simulink model "ofdm_txrx_mimo_2x"  (Simulink 6.6) (continued)
== system ofdm_txrx_mimo_2x/EDK Processor == (continued)
      | 
      | ps = xl_and(ps1Reg, aValidReg);
      | 
      | % ===== addrAck =====
      | 
      | % register ps
      | persistent psReg, psReg = xl_state(0, ps);
      | 
      | addrAck = xfix({xlUnsigned, 1, 0}, xl_and(xl_not(plbRstReg_), ps, xl_not(psReg)));
      | 
      | psReg = ps;
      | 
      | % ===== rdComp, rd/wr DAck =====
      |  
      | rdComp1 = xfix({xlUnsigned, 1, 0}, xl_and(addrAck, RNWReg));
      | 
      | NUM_rdCompDelay = 3;
      | persistent rdCompDelay, rdCompDelay = xl_state(zeros(1, NUM_rdCompDelay), rdComp1, NUM_rdCompDelay);
      | rdComp2 = rdCompDelay.back;
      | rdCompDelay.push_front_pop_back(rdComp1);
      | 
      | persistent rdCompReg, rdCompReg = xl_state(0, rdComp1);
      | rdComp = rdCompReg;
      | rdCompReg = rdComp2;
      | 
      | persistent rdDAckReg, rdDAckReg = xl_state(0, rdComp1);
      | rdDAck = rdDAckReg;
      | rdDAckReg = rdComp;
      | 
      | persistent wrDAckReg, wrDAckReg = xl_state(0, addrAck);
      | wrDAck = wrDAckReg;
      | wrDAckReg = xl_and(addrAck, xl_not(RNWReg));
      | 
      | % ===== rdDBus =====
      | 
      | rdSel = xl_or(rdComp2, rdComp);
      | 
      | if rdSel == 1
      |     rdDBus1 = rdData;
      | else
      |     rdDBus1 = 0;
      | end % if
      | 
      | persistent rdDBusReg, rdDBusReg = xl_state(0, rdDBus1);
      | rdDBus = rdDBusReg;
      | rdDBusReg = rdDBus1;
      | 
      | % rdDBus = xl_concat(rdDBus32, rdDBus32);
      | % rdDBus = rdDBus32;
      | 
      | % ===== update the persistent variables =====
      | 
      | plbRstReg_ = plbRst;
      | plbABusReg_ = plbABus;
      | plbPAValidReg_ = plbPAValid;
      | plbRNWReg_ = plbRNW;
      | plbWrDBusReg_ = xl_slice(plbWrDBus, DBUS_LEN-1, 0);
      settings: block_version=10.1.3
  plb_memmap : xbsIndex_r4/MCode  mfname=xlmax  explicit_period=off  period=1  dbl_ovrd=off  enable_stdout=off  enable_debug=off  mfilecontent=|  suppress_output=1  defparams={}  hide_port_list={}  block_type=mcode
      | function [read_bank_out, sm_Rx_Constellation_Scaling_w_din, sm_Rx_Constellation_Scaling_w_en, sm_Rx_ControlBits_w_din, sm_Rx_ControlBits_w_en, sm_Rx_FreqOffFilt_B_KI_w_din, sm_Rx_FreqOffFilt_B_KI_w_en, sm_Rx_FreqOffFilt_B_KP_w_din, sm_Rx_FreqOffFilt_B_KP_w_en, sm_Rx_OFDM_SymbolCounts_w_din, sm_Rx_OFDM_SymbolCounts_w_en, sm_Rx_PhaseNoiseTrack_K_w_din, sm_Rx_PhaseNoiseTrack_K_w_en, sm_Rx_PktDet_Delay_w_din, sm_Rx_PktDet_Delay_w_en, sm_Rx_PktDet_LongCorr_Params_w_din, sm_Rx_PktDet_LongCorr_Params_w_en, sm_Rx_pktByteNums_w_din, sm_Rx_pktByteNums_w_en, sm_Rx_pktDet_Tresholds_w_din, sm_Rx_pktDet_Tresholds_w_en, sm_TxRx_FFT_Scaling_w_din, sm_TxRx_FFT_Scaling_w_en, sm_TxRx_Interrupt_PktBuf_Ctrl_w_din, sm_TxRx_Interrupt_PktBuf_Ctrl_w_en, sm_Tx_ControlBits_w_din, sm_Tx_ControlBits_w_en, sm_Tx_OFDM_SymCounts_w_din, sm_Tx_OFDM_SymCounts_w_en, sm_Tx_Pilots_Index1_w_din, sm_Tx_Pilots_Index1_w_en, sm_Tx_Pilots_Index2_w_din, sm_Tx_Pilots_Index2_w_en, sm_Tx_Pilots_Value1_w_din, sm_Tx_Pilots_Value1_w_en, sm_Tx_Pilots_Value2_w_din, sm_Tx_Pilots_Value2_w_en, sm_Tx_PreambleMasking_w_din, sm_Tx_PreambleMasking_w_en, sm_Tx_PreambleScaling_w_din, sm_Tx_PreambleScaling_w_en, sm_Tx_Start_Reset_Control_w_din, sm_Tx_Start_Reset_Control_w_en, sm_RxModulation_addr, sm_RxModulation_din, sm_RxModulation_we, sm_TxModulation_addr, sm_TxModulation_din, sm_TxModulation_we] = plb_memmap(wrDBus, bankAddr, linearAddr, RNWReg, addrAck, sm_Rx_BER_Errors, sm_Rx_BER_TotalBits, sm_Rx_ControlBits_r, sm_Rx_Gains, sm_Rx_OFDM_SymbolCounts_r, sm_Rx_PktDet_Delay_r, sm_Rx_PktDet_LongCorr_Params_r, sm_Rx_pktByteNums_r, sm_Rx_pktDone_interruptStatus, sm_TxRx_Interrupt_PktBuf_Ctrl_r, sm_Tx_ControlBits_r, sm_Tx_OFDM_SymCounts_r, sm_Tx_PktRunning, sm_Tx_Start_Reset_Control_r, sm_RxModulation, sm_TxModulation)
      | 
      | 
      | % connvert the input data to UFix_32_0 (the bus data type)
      | % 'From Register' blocks
      | % sm_Rx_BER_Errors_bus = xfix({xlUnsigned, 32, 0}, 0);
      | sm_Rx_BER_Errors_bus = xl_force(sm_Rx_BER_Errors, xlUnsigned, 0);
      | 
      | % sm_Rx_BER_TotalBits_bus = xfix({xlUnsigned, 32, 0}, 0);
      | sm_Rx_BER_TotalBits_bus = xl_force(sm_Rx_BER_TotalBits, xlUnsigned, 0);
      | 
      | % sm_Rx_ControlBits_r_bus = xfix({xlUnsigned, 32, 0}, 0);
      | sm_Rx_ControlBits_r_bus = xl_force(sm_Rx_ControlBits_r, xlUnsigned, 0);
      | 
      | % sm_Rx_Gains_bus = xfix({xlUnsigned, 32, 0}, 0);
      | sm_Rx_Gains_bus = xl_force(sm_Rx_Gains, xlUnsigned, 0);
      | 
      | % sm_Rx_OFDM_SymbolCounts_r_bus = xfix({xlUnsigned, 32, 0}, 0);
      | sm_Rx_OFDM_SymbolCounts_r_bus = xl_force(sm_Rx_OFDM_SymbolCounts_r, xlUnsigned, 0);
      | 
      | % sm_Rx_PktDet_Delay_r_bus = xfix({xlUnsigned, 32, 0}, 0);
      | sm_Rx_PktDet_Delay_r_bus = xl_force(sm_Rx_PktDet_Delay_r, xlUnsigned, 0);
      | 
      | % sm_Rx_PktDet_LongCorr_Params_r_bus = xfix({xlUnsigned, 32, 0}, 0);
      | sm_Rx_PktDet_LongCorr_Params_r_bus = xl_force(sm_Rx_PktDet_LongCorr_Params_r, xlUnsigned, 0);
      | 
      | % sm_Rx_pktByteNums_r_bus = xfix({xlUnsigned, 32, 0}, 0);
      | sm_Rx_pktByteNums_r_bus = xl_force(sm_Rx_pktByteNums_r, xlUnsigned, 0);
      | 
      | % sm_Rx_pktDone_interruptStatus_bus = xfix({xlUnsigned, 32, 0}, 0);
      | sm_Rx_pktDone_interruptStatus_bus = xl_force(sm_Rx_pktDone_interruptStatus, xlUnsigned, 0);
      | 
      | % sm_TxRx_Interrupt_PktBuf_Ctrl_r_bus = xfix({xlUnsigned, 32, 0}, 0);
      | sm_TxRx_Interrupt_PktBuf_Ctrl_r_bus = xl_force(sm_TxRx_Interrupt_PktBuf_Ctrl_r, xlUnsigned, 0);
      | 
      | % sm_Tx_ControlBits_r_bus = xfix({xlUnsigned, 32, 0}, 0);
      | sm_Tx_ControlBits_r_bus = xl_force(sm_Tx_ControlBits_r, xlUnsigned, 0);
      | 
      | % sm_Tx_OFDM_SymCounts_r_bus = xfix({xlUnsigned, 32, 0}, 0);
      | sm_Tx_OFDM_SymCounts_r_bus = xl_force(sm_Tx_OFDM_SymCounts_r, xlUnsigned, 0);
      | 
      | % sm_Tx_PktRunning_bus = xfix({xlUnsigned, 32, 0}, 0);
      | sm_Tx_PktRunning_bus = xl_force(sm_Tx_PktRunning, xlUnsigned, 0);
      | 
      | % sm_Tx_Start_Reset_Control_r_bus = xfix({xlUnsigned, 32, 0}, 0);
      | sm_Tx_Start_Reset_Control_r_bus = xl_force(sm_Tx_Start_Reset_Control_r, xlUnsigned, 0);
      | 
      | % 'To Register' blocks
      | 
      | % 'From FIFO' blocks
      | % 'To FIFO' blocks
      | % 'Shared Memory' blocks
      | % RxModulation_bus = xfix({xlUnsigned, 32, 0}, 0);
      | sm_RxModulation_bus = xl_force(sm_RxModulation, xlUnsigned, 0);
      | 
      | % TxModulation_bus = xfix({xlUnsigned, 32, 0}, 0);
      | sm_TxModulation_bus = xl_force(sm_TxModulation, xlUnsigned, 0);
      | 
      | 
      | % 'dout' ports of 'From Register' blocks
      | 
      | % registered register mux output
      | persistent reg_bank_out_reg; reg_bank_out_reg = xl_state(0, {xlUnsigned, 32, 0});
      | reg_bank_out = reg_bank_out_reg;
      | 
      | if linearAddr == 0
      |     reg_bank_out_reg = sm_Rx_BER_Errors_bus;
      | elseif linearAddr == 1
      |     reg_bank_out_reg = sm_Rx_BER_TotalBits_bus;
      | elseif linearAddr == 2
      |     reg_bank_out_reg = sm_Rx_ControlBits_r_bus;
      | elseif linearAddr == 3
      |     reg_bank_out_reg = sm_Rx_Gains_bus;
      | elseif linearAddr == 4
      |     reg_bank_out_reg = sm_Rx_OFDM_SymbolCounts_r_bus;
      | elseif linearAddr == 5
      |     reg_bank_out_reg = sm_Rx_PktDet_Delay_r_bus;
      | elseif linearAddr == 6
      |     reg_bank_out_reg = sm_Rx_PktDet_LongCorr_Params_r_bus;
      | elseif linearAddr == 7
      |     reg_bank_out_reg = sm_Rx_pktByteNums_r_bus;
      | elseif linearAddr == 8
      |     reg_bank_out_reg = sm_Rx_pktDone_interruptStatus_bus;
      | elseif linearAddr == 9
      |     reg_bank_out_reg = sm_TxRx_Interrupt_PktBuf_Ctrl_r_bus;
      | elseif linearAddr == 10
      |     reg_bank_out_reg = sm_Tx_ControlBits_r_bus;
      | elseif linearAddr == 11
      |     reg_bank_out_reg = sm_Tx_OFDM_SymCounts_r_bus;
      | elseif linearAddr == 12
      |     reg_bank_out_reg = sm_Tx_PktRunning_bus;
      | elseif linearAddr == 13
      |     reg_bank_out_reg = sm_Tx_Start_Reset_Control_r_bus;
      | 
      | end
      | 
      | 
      | % 'From FIFO' and 'To FIFO' blocks
      | 
      | 
      | 
      | 
      | 
      | opCode = xl_concat(addrAck, RNWReg, bankAddr, linearAddr);
      | 
      | % 'Shared Memory' blocks
      | 
      | sm_RxModulation_sel_value = xl_concat(xl_slice(linearAddr, ...
      |                                         xl_nbits(linearAddr) - 1, ...
      |                                         8) ...
      |                                     );
      | if sm_RxModulation_sel_value == xfix({xlUnsigned, ...
      |                                         xl_nbits(linearAddr) - 8, ...
      |                                         0}, ...
      |                                         0);
      |     sm_RxModulation_sel = true;
      | else
      |     sm_RxModulation_sel = false;
      | end
      | sm_TxModulation_sel_value = xl_concat(xl_slice(linearAddr, ...
      |                                         xl_nbits(linearAddr) - 1, ...
      |                                         8) ...
      |                                     );
      | if sm_TxModulation_sel_value == xfix({xlUnsigned, ...
      |                                         xl_nbits(linearAddr) - 8, ...
      |                                         0}, ...
      |                                         1);
      |     sm_TxModulation_sel = true;
      | else
      |     sm_TxModulation_sel = false;
      | end
      | 
      | 
      | % registered Shared Memory mux output
      | persistent ram_bank_out_reg; ram_bank_out_reg = xl_state(0, {xlUnsigned, 32, 0});
      | ram_bank_out = ram_bank_out_reg;
      | if sm_RxModulation_sel
      |     ram_bank_out_reg = sm_RxModulation_bus;
      | elseif sm_TxModulation_sel
      |     ram_bank_out_reg = sm_TxModulation_bus;
      | end
      | 
      | % 'din' ports of 'Shared Memory' blocks
      | sm_RxModulation_din = xl_force(xl_slice(wrDBus, 4 - 1, 0), ...
      |                                  xlUnsigned, ...
      |                                  0);
      | sm_TxModulation_din = xl_force(xl_slice(wrDBus, 4 - 1, 0), ...
      |                                  xlUnsigned, ...
      |                                  0);
      | 
      | 
      | % 'we' ports of 'Shared Memory' blocks
      | persistent sm_RxModulation_we_reg; sm_RxModulation_we_reg = xl_state(false, {xlBoolean});
      | sm_RxModulation_we = sm_RxModulation_we_reg;
      | opCode_sm_RxModulation = xl_concat(addrAck, ...
      |                                      RNWReg, ...
      |                                      bankAddr, ...
      |                                      xl_slice(linearAddr, ...
      |                                               xl_nbits(linearAddr) - 1, ...
      |                                               8) ...
      |                                     );
      | if opCode_sm_RxModulation == xl_concat(xfix({xlUnsigned, 4, 0}, 8), ...
      |                                          xfix({xlUnsigned, ...
      |                                                xl_nbits(linearAddr) - 8, ...
      |                                                0}, ...
      |                                                0) ...
      |                                         );
      |     sm_RxModulation_we_reg = true;
      | else
      |     sm_RxModulation_we_reg = false;
      | end
      | persistent sm_TxModulation_we_reg; sm_TxModulation_we_reg = xl_state(false, {xlBoolean});
      | sm_TxModulation_we = sm_TxModulation_we_reg;
      | opCode_sm_TxModulation = xl_concat(addrAck, ...
      |                                      RNWReg, ...
      |                                      bankAddr, ...
      |                                      xl_slice(linearAddr, ...
      |                                               xl_nbits(linearAddr) - 1, ...
      |                                               8) ...
      |                                     );
      | if opCode_sm_TxModulation == xl_concat(xfix({xlUnsigned, 4, 0}, 8), ...
      |                                          xfix({xlUnsigned, ...
      |                                                xl_nbits(linearAddr) - 8, ...
      |                                                0}, ...
      |                                                1) ...
      |                                         );
      |     sm_TxModulation_we_reg = true;
      | else
      |     sm_TxModulation_we_reg = false;
      | end
      | 
      | 
      | % 'addr' ports of 'Shared Memory' blocks
      | persistent sm_RxModulation_addr_reg; 
      | sm_RxModulation_addr_reg = xl_state(0, {xlUnsigned, 8, 0});
      | sm_RxModulation_addr = sm_RxModulation_addr_reg;
      | if addrAck == 1
      |     sm_RxModulation_addr_reg = xl_slice(linearAddr, 8, 0);
      | else
      |     sm_RxModulation_addr_reg = sm_RxModulation_addr_reg;
      | end
      | persistent sm_TxModulation_addr_reg; 
      | sm_TxModulation_addr_reg = xl_state(0, {xlUnsigned, 8, 0});
      | sm_TxModulation_addr = sm_TxModulation_addr_reg;
      | if addrAck == 1
      |     sm_TxModulation_addr_reg = xl_slice(linearAddr, 8, 0);
      | else
      |     sm_TxModulation_addr_reg = sm_TxModulation_addr_reg;
      | end
      | 
      | 
      | % 're' ports of 'From FIFO' blocks
      | 
      | 
      | % 'en' ports of 'To Register' blocks
      | if opCode == xl_concat(xfix({xlUnsigned, 4, 0}, 10), ...
      |                        xfix({xlUnsigned, xl_nbits(linearAddr), 0}, 0))
      |     sm_Rx_Constellation_Scaling_w_en = true;
      | else
      |     sm_Rx_Constellation_Scaling_w_en = false;
      | end
      | if opCode == xl_concat(xfix({xlUnsigned, 4, 0}, 10), ...
      |                        xfix({xlUnsigned, xl_nbits(linearAddr), 0}, 1))
      |     sm_Rx_ControlBits_w_en = true;
      | else
      |     sm_Rx_ControlBits_w_en = false;
      | end
      | if opCode == xl_concat(xfix({xlUnsigned, 4, 0}, 10), ...
      |                        xfix({xlUnsigned, xl_nbits(linearAddr), 0}, 2))
      |     sm_Rx_FreqOffFilt_B_KI_w_en = true;
      | else
      |     sm_Rx_FreqOffFilt_B_KI_w_en = false;
      | end
      | if opCode == xl_concat(xfix({xlUnsigned, 4, 0}, 10), ...
      |                        xfix({xlUnsigned, xl_nbits(linearAddr), 0}, 3))
      |     sm_Rx_FreqOffFilt_B_KP_w_en = true;
      | else
      |     sm_Rx_FreqOffFilt_B_KP_w_en = false;
      | end
      | if opCode == xl_concat(xfix({xlUnsigned, 4, 0}, 10), ...
      |                        xfix({xlUnsigned, xl_nbits(linearAddr), 0}, 4))
      |     sm_Rx_OFDM_SymbolCounts_w_en = true;
      | else
      |     sm_Rx_OFDM_SymbolCounts_w_en = false;
      | end
      | if opCode == xl_concat(xfix({xlUnsigned, 4, 0}, 10), ...
      |                        xfix({xlUnsigned, xl_nbits(linearAddr), 0}, 5))
      |     sm_Rx_PhaseNoiseTrack_K_w_en = true;
      | else
      |     sm_Rx_PhaseNoiseTrack_K_w_en = false;
      | end
      | if opCode == xl_concat(xfix({xlUnsigned, 4, 0}, 10), ...
      |                        xfix({xlUnsigned, xl_nbits(linearAddr), 0}, 6))
      |     sm_Rx_PktDet_Delay_w_en = true;
      | else
      |     sm_Rx_PktDet_Delay_w_en = false;
      | end
      | if opCode == xl_concat(xfix({xlUnsigned, 4, 0}, 10), ...
      |                        xfix({xlUnsigned, xl_nbits(linearAddr), 0}, 7))
      |     sm_Rx_PktDet_LongCorr_Params_w_en = true;
      | else
      |     sm_Rx_PktDet_LongCorr_Params_w_en = false;
      | end
      | if opCode == xl_concat(xfix({xlUnsigned, 4, 0}, 10), ...
      |                        xfix({xlUnsigned, xl_nbits(linearAddr), 0}, 8))
      |     sm_Rx_pktByteNums_w_en = true;
      | else
      |     sm_Rx_pktByteNums_w_en = false;
      | end
      | if opCode == xl_concat(xfix({xlUnsigned, 4, 0}, 10), ...
      |                        xfix({xlUnsigned, xl_nbits(linearAddr), 0}, 9))
      |     sm_Rx_pktDet_Tresholds_w_en = true;
      | else
      |     sm_Rx_pktDet_Tresholds_w_en = false;
      | end
      | if opCode == xl_concat(xfix({xlUnsigned, 4, 0}, 10), ...
      |                        xfix({xlUnsigned, xl_nbits(linearAddr), 0}, 10))
      |     sm_TxRx_FFT_Scaling_w_en = true;
      | else
      |     sm_TxRx_FFT_Scaling_w_en = false;
      | end
      | if opCode == xl_concat(xfix({xlUnsigned, 4, 0}, 10), ...
      |                        xfix({xlUnsigned, xl_nbits(linearAddr), 0}, 11))
      |     sm_TxRx_Interrupt_PktBuf_Ctrl_w_en = true;
      | else
      |     sm_TxRx_Interrupt_PktBuf_Ctrl_w_en = false;
      | end
      | if opCode == xl_concat(xfix({xlUnsigned, 4, 0}, 10), ...
      |                        xfix({xlUnsigned, xl_nbits(linearAddr), 0}, 12))
      |     sm_Tx_ControlBits_w_en = true;
      | else
      |     sm_Tx_ControlBits_w_en = false;
      | end
      | if opCode == xl_concat(xfix({xlUnsigned, 4, 0}, 10), ...
      |                        xfix({xlUnsigned, xl_nbits(linearAddr), 0}, 13))
      |     sm_Tx_OFDM_SymCounts_w_en = true;
      | else
      |     sm_Tx_OFDM_SymCounts_w_en = false;
      | end
      | if opCode == xl_concat(xfix({xlUnsigned, 4, 0}, 10), ...
      |                        xfix({xlUnsigned, xl_nbits(linearAddr), 0}, 14))
      |     sm_Tx_Pilots_Index1_w_en = true;
      | else
      |     sm_Tx_Pilots_Index1_w_en = false;
      | end
      | if opCode == xl_concat(xfix({xlUnsigned, 4, 0}, 10), ...
      |                        xfix({xlUnsigned, xl_nbits(linearAddr), 0}, 15))
      |     sm_Tx_Pilots_Index2_w_en = true;
      | else
      |     sm_Tx_Pilots_Index2_w_en = false;
      | end
      | if opCode == xl_concat(xfix({xlUnsigned, 4, 0}, 10), ...
      |                        xfix({xlUnsigned, xl_nbits(linearAddr), 0}, 16))
      |     sm_Tx_Pilots_Value1_w_en = true;
      | else
      |     sm_Tx_Pilots_Value1_w_en = false;
      | end
      | if opCode == xl_concat(xfix({xlUnsigned, 4, 0}, 10), ...
      |                        xfix({xlUnsigned, xl_nbits(linearAddr), 0}, 17))
      |     sm_Tx_Pilots_Value2_w_en = true;
      | else
      |     sm_Tx_Pilots_Value2_w_en = false;
      | end
      | if opCode == xl_concat(xfix({xlUnsigned, 4, 0}, 10), ...
      |                        xfix({xlUnsigned, xl_nbits(linearAddr), 0}, 18))
      |     sm_Tx_PreambleMasking_w_en = true;
      | else
      |     sm_Tx_PreambleMasking_w_en = false;
      | end
      | if opCode == xl_concat(xfix({xlUnsigned, 4, 0}, 10), ...
      |                        xfix({xlUnsigned, xl_nbits(linearAddr), 0}, 19))
      |     sm_Tx_PreambleScaling_w_en = true;
      | else
      |     sm_Tx_PreambleScaling_w_en = false;
      | end
      | if opCode == xl_concat(xfix({xlUnsigned, 4, 0}, 10), ...
      |                        xfix({xlUnsigned, xl_nbits(linearAddr), 0}, 20))
      |     sm_Tx_Start_Reset_Control_w_en = true;
      | else
      |     sm_Tx_Start_Reset_Control_w_en = false;
      | end
      | 
      | 
      | % 'din' ports of 'To FIFO' blocks
      | 
      | 
      | % 'we' ports of 'To FIFO' blocks
      | 
      | 
      | % 'din' ports of 'To Register' blocks
      | sm_Rx_Constellation_Scaling_w_din = xl_force(xl_slice(wrDBus, 32 - 1, 0), ...
      |                                  xlUnsigned, ...
      |                                  0);
      | sm_Rx_ControlBits_w_din = xl_force(xl_slice(wrDBus, 32 - 1, 0), ...
      |                                  xlUnsigned, ...
      |                                  0);
      | sm_Rx_FreqOffFilt_B_KI_w_din = xl_force(xl_slice(wrDBus, 32 - 1, 0), ...
      |                                  xlUnsigned, ...
      |                                  0);
      | sm_Rx_FreqOffFilt_B_KP_w_din = xl_force(xl_slice(wrDBus, 32 - 1, 0), ...
      |                                  xlUnsigned, ...
      |                                  0);
      | sm_Rx_OFDM_SymbolCounts_w_din = xl_force(xl_slice(wrDBus, 32 - 1, 0), ...
      |                                  xlUnsigned, ...
      |                                  0);
      | sm_Rx_PhaseNoiseTrack_K_w_din = xl_force(xl_slice(wrDBus, 32 - 1, 0), ...
      |                                  xlUnsigned, ...
      |                                  0);
      | sm_Rx_PktDet_Delay_w_din = xl_force(xl_slice(wrDBus, 32 - 1, 0), ...
      |                                  xlUnsigned, ...
      |                                  0);
      | sm_Rx_PktDet_LongCorr_Params_w_din = xl_force(xl_slice(wrDBus, 32 - 1, 0), ...
      |                                  xlUnsigned, ...
      |                                  0);
      | sm_Rx_pktByteNums_w_din = xl_force(xl_slice(wrDBus, 32 - 1, 0), ...
      |                                  xlUnsigned, ...
      |                                  0);
      | sm_Rx_pktDet_Tresholds_w_din = xl_force(xl_slice(wrDBus, 32 - 1, 0), ...
      |                                  xlUnsigned, ...
      |                                  0);
      | sm_TxRx_FFT_Scaling_w_din = xl_force(xl_slice(wrDBus, 12 - 1, 0), ...
      |                                  xlUnsigned, ...
      |                                  0);
      | sm_TxRx_Interrupt_PktBuf_Ctrl_w_din = xl_force(xl_slice(wrDBus, 32 - 1, 0), ...
      |                                  xlUnsigned, ...
      |                                  0);
      | sm_Tx_ControlBits_w_din = xl_force(xl_slice(wrDBus, 32 - 1, 0), ...
      |                                  xlUnsigned, ...
      |                                  0);
      | sm_Tx_OFDM_SymCounts_w_din = xl_force(xl_slice(wrDBus, 32 - 1, 0), ...
      |                                  xlUnsigned, ...
      |                                  0);
      | sm_Tx_Pilots_Index1_w_din = xl_force(xl_slice(wrDBus, 32 - 1, 0), ...
      |                                  xlUnsigned, ...
      |                                  0);
      | sm_Tx_Pilots_Index2_w_din = xl_force(xl_slice(wrDBus, 32 - 1, 0), ...
      |                                  xlUnsigned, ...
      |                                  0);
      | sm_Tx_Pilots_Value1_w_din = xl_force(xl_slice(wrDBus, 32 - 1, 0), ...
      |                                  xlUnsigned, ...
      |                                  0);
      | sm_Tx_Pilots_Value2_w_din = xl_force(xl_slice(wrDBus, 32 - 1, 0), ...
      |                                  xlUnsigned, ...
      |                                  0);
      | sm_Tx_PreambleMasking_w_din = xl_force(xl_slice(wrDBus, 32 - 1, 0), ...
      |                                  xlUnsigned, ...
      |                                  0);
      | sm_Tx_PreambleScaling_w_din = xl_force(xl_slice(wrDBus, 16 - 1, 0), ...
      |                                  xlUnsigned, ...
      |                                  0);
      | sm_Tx_Start_Reset_Control_w_din = xl_force(xl_slice(wrDBus, 32 - 1, 0), ...
      |                                  xlUnsigned, ...
      |                                  0);
      | 
      | 
      | persistent read_bank_out_reg; read_bank_out_reg = xl_state(0, {xlUnsigned, 32, 0});
      | read_bank_out = read_bank_out_reg;
      | 
      | persistent bankAddr_reg; bankAddr_reg = xl_state(0, bankAddr);
      | 
      | if bankAddr_reg == 0
      |     % Bank 0: Shared Memories
      |     read_bank_out_reg = ram_bank_out;
      | elseif bankAddr_reg == 1
      |     % Bank 1: From/To FIFOs
      |     read_bank_out_reg =  0;
      | elseif bankAddr_reg == 2
      |     % Bank 2: From/To Registers
      |     read_bank_out_reg = reg_bank_out;
      | elseif bankAddr_reg == 3
      |     % Bank 3: Configuration Registers
      |     read_bank_out_reg = 0;
      | end
      | 
      | bankAddr_reg = bankAddr;
      settings: block_version=10.1.3
  sg_plb_addrpref : xbsIndex_r4/Gateway In  arith_type=Unsigned  n_bits=19  bin_pt=0  quantization="Round  (unbiased: +/- Inf)"  overflow=Saturate  period=xlGetSimulinkPeriod(gcb)  dbl_ovrd=off  timing_constraint=None  locs_specified=off  LOCs={}  block_type=gatewayin
      settings: infoedit="Gateway in block.  Converts inputs of type Simulink integer, double and fixed point to  Xilinx fixed point type.<P><P>Hardware notes:  In hardware these blocks become top level input ports."  sginterface={'Xilinx'=>{'jtaghwcosim'=>{'non_memory_mapped_port'=>'sg_plb_addrpref'}},'iopad'=>{'constraint'=>'#'}}  block_version=10.1.3
  wires:
  plb_decode/2 -> plb_memmap/5, Sl_addrAck/1  "Sl_addrAck"
  plb_decode/4 -> Sl_wrDAck/1, Sl_wrComp/1  "Sl_wrDAck"
  plb_memmap/49 -> Shared Memory1/3  "TxModulation_we"
  plb_memmap/48 -> Shared Memory1/2  "TxModulation_din"
  plb_memmap/47 -> Shared Memory1/1  "TxModulation_addr"
  plb_memmap/46 -> Shared Memory/3  "RxModulation_we"
  plb_memmap/45 -> Shared Memory/2  "RxModulation_din"
  plb_memmap/44 -> Shared Memory/1  "RxModulation_addr"
  plb_memmap/43 -> To Register20/2  "Tx_Start_Reset_Control_w_en"
  plb_memmap/42 -> To Register20/1  "Tx_Start_Reset_Control_w_din"
  plb_memmap/41 -> To Register19/2  "Tx_PreambleScaling_w_en"
  plb_memmap/40 -> To Register19/1  "Tx_PreambleScaling_w_din"
  plb_memmap/39 -> To Register18/2  "Tx_PreambleMasking_w_en"
  plb_memmap/38 -> To Register18/1  "Tx_PreambleMasking_w_din"
  plb_memmap/37 -> To Register17/2  "Tx_Pilots_Value2_w_en"
  plb_memmap/36 -> To Register17/1  "Tx_Pilots_Value2_w_din"
  plb_memmap/35 -> To Register16/2  "Tx_Pilots_Value1_w_en"
  plb_memmap/34 -> To Register16/1  "Tx_Pilots_Value1_w_din"
  plb_memmap/33 -> To Register15/2  "Tx_Pilots_Index2_w_en"
  plb_memmap/32 -> To Register15/1  "Tx_Pilots_Index2_w_din"
  plb_memmap/31 -> To Register14/2  "Tx_Pilots_Index1_w_en"
  plb_memmap/30 -> To Register14/1  "Tx_Pilots_Index1_w_din"
  plb_memmap/29 -> To Register13/2  "Tx_OFDM_SymCounts_w_en"
  plb_memmap/28 -> To Register13/1  "Tx_OFDM_SymCounts_w_din"
  plb_memmap/27 -> To Register12/2  "Tx_ControlBits_w_en"
  plb_memmap/26 -> To Register12/1  "Tx_ControlBits_w_din"
  plb_memmap/25 -> To Register11/2  "TxRx_Interrupt_PktBuf_Ctrl_w_en"
  plb_memmap/24 -> To Register11/1  "TxRx_Interrupt_PktBuf_Ctrl_w_din"
  plb_memmap/23 -> To Register10/2  "TxRx_FFT_Scaling_w_en"
  plb_memmap/22 -> To Register10/1  "TxRx_FFT_Scaling_w_din"
  plb_memmap/21 -> To Register9/2  "Rx_pktDet_Tresholds_w_en"
  plb_memmap/20 -> To Register9/1  "Rx_pktDet_Tresholds_w_din"
  plb_memmap/19 -> To Register8/2  "Rx_pktByteNums_w_en"
  plb_memmap/18 -> To Register8/1  "Rx_pktByteNums_w_din"
  plb_memmap/17 -> To Register7/2  "Rx_PktDet_LongCorr_Params_w_en"
  plb_memmap/16 -> To Register7/1  "Rx_PktDet_LongCorr_Params_w_din"
  plb_memmap/15 -> To Register6/2  "Rx_PktDet_Delay_w_en"
  plb_memmap/14 -> To Register6/1  "Rx_PktDet_Delay_w_din"
  plb_memmap/13 -> To Register5/2  "Rx_PhaseNoiseTrack_K_w_en"
  plb_memmap/12 -> To Register5/1  "Rx_PhaseNoiseTrack_K_w_din"
  plb_memmap/11 -> To Register4/2  "Rx_OFDM_SymbolCounts_w_en"
  plb_memmap/10 -> To Register4/1  "Rx_OFDM_SymbolCounts_w_din"
  plb_memmap/9 -> To Register3/2  "Rx_FreqOffFilt_B_KP_w_en"
  plb_memmap/8 -> To Register3/1  "Rx_FreqOffFilt_B_KP_w_din"
  plb_memmap/7 -> To Register2/2  "Rx_FreqOffFilt_B_KI_w_en"
  plb_memmap/6 -> To Register2/1  "Rx_FreqOffFilt_B_KI_w_din"
  plb_memmap/5 -> To Register1/2  "Rx_ControlBits_w_en"
  plb_memmap/4 -> To Register1/1  "Rx_ControlBits_w_din"
  plb_memmap/3 -> To Register/2  "Rx_Constellation_Scaling_w_en"
  plb_memmap/2 -> To Register/1  "Rx_Constellation_Scaling_w_din"
  plb_memmap/1 -> plb_decode/6  "rdData"
  Shared Memory1/1 -> plb_memmap/21  "TxModulation_dout"
  Shared Memory/1 -> plb_memmap/20  "RxModulation_dout"
  From Register13/1 -> plb_memmap/19  "Tx_Start_Reset_Control_r_dout"
  From Register12/1 -> plb_memmap/18  "Tx_PktRunning_dout"
  From Register11/1 -> plb_memmap/17  "Tx_OFDM_SymCounts_r_dout"
  From Register10/1 -> plb_memmap/16  "Tx_ControlBits_r_dout"
  From Register9/1 -> plb_memmap/15  "TxRx_Interrupt_PktBuf_Ctrl_r_dout"
  From Register8/1 -> plb_memmap/14  "Rx_pktDone_interruptStatus_dout"
  From Register7/1 -> plb_memmap/13  "Rx_pktByteNums_r_dout"
  From Register6/1 -> plb_memmap/12  "Rx_PktDet_LongCorr_Params_r_dout"
  From Register5/1 -> plb_memmap/11  "Rx_PktDet_Delay_r_dout"
  From Register4/1 -> plb_memmap/10  "Rx_OFDM_SymbolCounts_r_dout"
  From Register3/1 -> plb_memmap/9  "Rx_Gains_dout"
  From Register2/1 -> plb_memmap/8  "Rx_ControlBits_r_dout"
  From Register1/1 -> plb_memmap/7  "Rx_BER_TotalBits_dout"
  From Register/1 -> plb_memmap/6  "Rx_BER_Errors_dout"
  plb_decode/6 -> plb_memmap/4  "RNWReg"
  plb_decode/9 -> plb_memmap/3  "linearAddr"
  plb_decode/5 -> plb_memmap/2  "bankAddr"
  plb_decode/1 -> plb_memmap/1  "wrDBusReg"
  To Register20/1 -> Terminator27/1  "Tx_Start_Reset_Control_w_dout"
  To Register19/1 -> Terminator26/1  "Tx_PreambleScaling_w_dout"
  To Register18/1 -> Terminator25/1  "Tx_PreambleMasking_w_dout"
  To Register17/1 -> Terminator24/1  "Tx_Pilots_Value2_w_dout"
  To Register16/1 -> Terminator23/1  "Tx_Pilots_Value1_w_dout"
  To Register15/1 -> Terminator22/1  "Tx_Pilots_Index2_w_dout"
  To Register14/1 -> Terminator21/1  "Tx_Pilots_Index1_w_dout"
  To Register13/1 -> Terminator20/1  "Tx_OFDM_SymCounts_w_dout"
  To Register12/1 -> Terminator19/1  "Tx_ControlBits_w_dout"
  To Register11/1 -> Terminator18/1  "TxRx_Interrupt_PktBuf_Ctrl_w_dout"
  To Register10/1 -> Terminator17/1  "TxRx_FFT_Scaling_w_dout"
  To Register9/1 -> Terminator16/1  "Rx_pktDet_Tresholds_w_dout"
  To Register8/1 -> Terminator15/1  "Rx_pktByteNums_w_dout"
  To Register7/1 -> Terminator14/1  "Rx_PktDet_LongCorr_Params_w_dout"
  To Register6/1 -> Terminator13/1  "Rx_PktDet_Delay_w_dout"
  To Register5/1 -> Terminator12/1  "Rx_PhaseNoiseTrack_K_w_dout"
  To Register4/1 -> Terminator11/1  "Rx_OFDM_SymbolCounts_w_dout"
  To Register3/1 -> Terminator10/1  "Rx_FreqOffFilt_B_KP_w_dout"
  To Register2/1 -> Terminator9/1  "Rx_FreqOffFilt_B_KI_w_dout"
  To Register1/1 -> Terminator8/1  "Rx_ControlBits_w_dout"
  To Register/1 -> Terminator7/1  "Rx_Constellation_Scaling_w_dout"
  plb_decode/8 -> Sl_rdDBus/1  "Sl_rdDBus"
  plb_decode/7 -> Sl_rdDAck/1  "Sl_rdDAck"
  plb_decode/3 -> Sl_rdComp/1  "Sl_rdComp"
  sg_plb_addrpref/1 -> plb_decode/7  "addrPref"
  PLB_wrDBus/1 -> plb_decode/5  "PLB_wrDBus"
  PLB_RNW/1 -> plb_decode/4  "PLB_RNW"
  PLB_PAValid/1 -> plb_decode/3  "PLB_PAValid"
  PLB_ABus/1 -> plb_decode/2  "PLB_ABus"
  SPLB_Rst/1 -> plb_decode/1  "SPLB_Rst"
  Constant6/1 -> sg_plb_addrpref/1
  Constant5/1 -> Sl_wait/1  "Sl_wait"
  Constant4/1 -> PLB_wrDBus/1
  Constant3/1 -> PLB_RNW/1
  Constant2/1 -> PLB_PAValid/1
  Constant1/1 -> PLB_ABus/1
  Constant/1 -> SPLB_Rst/1
  Sl_wrComp/1 -> Terminator6/1
  Sl_wrDAck/1 -> Terminator5/1
  Sl_wait/1 -> Terminator4/1
  Sl_rdDBus/1 -> Terminator3/1
  Sl_rdDAck/1 -> Terminator2/1
  Sl_rdComp/1 -> Terminator1/1
  Sl_addrAck/1 -> Terminator/1
